simulink model "HvacFrntOutSurrndgsTOutl"  (R2022b)
settings: Solver=FixedStepDiscrete  StartTime=0.0  StopTime=10.0  FixedStep=0.2  RelTol=1e-3
settings Model: Creator=blue.ge  LastModifiedBy=blue.ge
settings DataIOCC: SaveFormat=StructureWithTime
settings OptimizationCC: DefaultParameterBehavior=Inlined  EnhancedBackFolding=on  PassReuseOutputArgsAs=Individual arguments  ZeroExternalMemoryAtStartup=off  ZeroInternalMemoryAtStartup=off  OptimizeBlockOrder=speed  DifferentSizesBufferReuse=on  OptimizationCustomize=off  EfficientTunableParamExpr=on
settings DebuggingCC: ParameterTunabilityLossMsg=error
settings ModelReferenceCC: ModelReferenceNumInstancesAllowed=Single
settings SFSimCC: MATLABDynamicMemAlloc=off
settings RTWCC: Description=AUTOSAR  SystemTargetFile=autosar.tlc  GenCodeOnly=on  TemplateMakefile=ert_default_tmf
settings CodeAppCC: ForceParamTrailComments=on  IgnoreCustomStorageClasses=off  OperatorAnnotations=on  SimulinkDataObjDesc=on  SFDataObjDesc=on  InsertBlockDesc=on
settings STFCustomTargetCC: TargetLangStandard=C99 (ISO)  MultiwordLength=256  ParMdlRefBuildCompliant=on  CompOptLevelCompliant=on  IncludeMdlTerminateFcn=off  CombineOutputUpdateFcns=on  SuppressErrorStatus=on  ERTCustomFileBanners=on  SupportNonFinite=off  SupportComplex=off  SupportContinuousTime=off  SupportNonInlinedSFcns=off  RemoveResetFunc=on  MATLABClassNameForMDSCustomization=Simulink.SoftwareTarget.ERTCustomization  AutosarCompliant=on  UseToolchainInfoCompliant=on  LookupTableObjectStructAxisOrder=2,1,3,4,...  LUTObjectStructOrderEvenSpacing=Size,Table,Breakpoints  SystemTargetFile=autosar.tlc
settings ERTTargetCC: TargetLangStandard=C99 (ISO)  ConcurrentExecutionCompliant=off  IncludeMdlTerminateFcn=off  SuppressErrorStatus=on  SupportNonFinite=off  SupportComplex=off  ModelStepFunctionPrototypeControlCompliant=off  CPPClassGenCompliant=off  AutosarCompliant=on  LookupTableObjectStructAxisOrder=2,1,3,4,...  LUTObjectStructOrderEvenSpacing=Size,Table,Breakpoints  InlinedParameterPlacement=NonHierarchical  ERTCustomFileTemplate=example_file_process.tlc

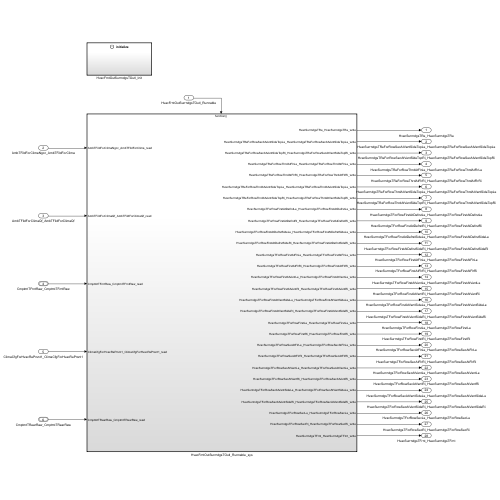
[thumbnail: Simulink's stored preview of the top-level diagram]
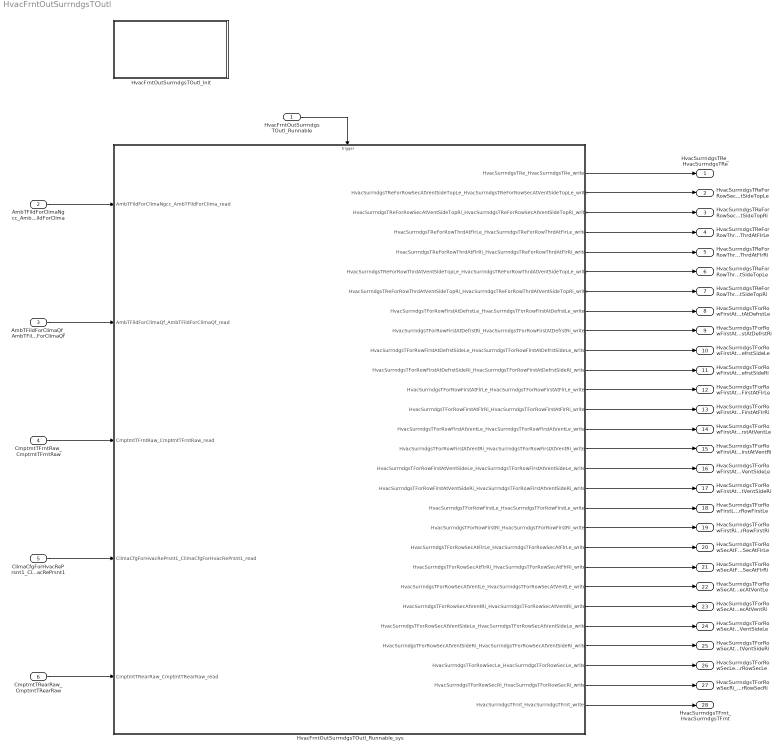
== system HvacFrntOutSurrndgsTOutl ==
  HvacFrntOutSurrndgsTOutl_Runnable : Inport  SampleTime=0.2  OutputFunctionCall=on
  AmbTFildForClimaNgcc_AmbTFildForClima : Inport  Port=2  OutDataTypeStr=T30  PortDimensions=1
  AmbTFildForClimaQf_AmbTFildForClimaQf : Inport  Port=3  OutDataTypeStr=Enum: GenQf1  PortDimensions=1
  CmptmtTFrntRaw_CmptmtTFrntRaw : Inport  Port=4  OutDataTypeStr=Bus: CmptmtTFrnt  PortDimensions=1  BusOutputAsStruct=on
  ClimaCfgForHvacRePrsnt1_ClimaCfgForHvacRePrsnt1 : Inport  Port=5  OutDataTypeStr=Enum: CarCfgForHvacRe  PortDimensions=1
  CmptmtTRearRaw_CmptmtTRearRaw : Inport  Port=6  OutDataTypeStr=Bus: CmptmtTFrnt  PortDimensions=1  BusOutputAsStruct=on
  HvacFrntOutSurrndgsTOutl_Init : Subsystem
  HvacFrntOutSurrndgsTOutl_Runnable_sys : Subsystem
  HvacSurrndgsTRe_HvacSurrndgsTRe : Outport  OutDataTypeStr=T30  PortDimensions=1
  HvacSurrndgsTReForRowSecAtVentSideTopLe_HvacSurrndgsTReForRowSecAtVentSideTopLe : Outport  Port=2  OutDataTypeStr=T30  PortDimensions=1
  HvacSurrndgsTReForRowSecAtVentSideTopRi_HvacSurrndgsTReForRowSecAtVentSideTopRi : Outport  Port=3  OutDataTypeStr=T30  PortDimensions=1
  HvacSurrndgsTReForRowThrdAtFlrLe_HvacSurrndgsTReForRowThrdAtFlrLe : Outport  Port=4  OutDataTypeStr=T30  PortDimensions=1
  HvacSurrndgsTReForRowThrdAtFlrRi_HvacSurrndgsTReForRowThrdAtFlrRi : Outport  Port=5  OutDataTypeStr=T30  PortDimensions=1
  HvacSurrndgsTReForRowThrdAtVentSideTopLe_HvacSurrndgsTReForRowThrdAtVentSideTopLe : Outport  Port=6  OutDataTypeStr=T30  PortDimensions=1
  HvacSurrndgsTReForRowThrdAtVentSideTopRi_HvacSurrndgsTReForRowThrdAtVentSideTopRi : Outport  Port=7  OutDataTypeStr=T30  PortDimensions=1
  HvacSurrndgsTForRowFirstAtDefrstLe_HvacSurrndgsTForRowFirstAtDefrstLe : Outport  Port=8  OutDataTypeStr=T30  PortDimensions=1
  HvacSurrndgsTForRowFirstAtDefrstRi_HvacSurrndgsTForRowFirstAtDefrstRi : Outport  Port=9  OutDataTypeStr=T30  PortDimensions=1
  HvacSurrndgsTForRowFirstAtDefrstSideLe_HvacSurrndgsTForRowFirstAtDefrstSideLe : Outport  Port=10  OutDataTypeStr=T30  PortDimensions=1
  HvacSurrndgsTForRowFirstAtDefrstSideRi_HvacSurrndgsTForRowFirstAtDefrstSideRi : Outport  Port=11  OutDataTypeStr=T30  PortDimensions=1
  HvacSurrndgsTForRowFirstAtFlrLe_HvacSurrndgsTForRowFirstAtFlrLe : Outport  Port=12  OutDataTypeStr=T30  PortDimensions=1
  HvacSurrndgsTForRowFirstAtFlrRi_HvacSurrndgsTForRowFirstAtFlrRi : Outport  Port=13  OutDataTypeStr=T30  PortDimensions=1
  HvacSurrndgsTForRowFirstAtVentLe_HvacSurrndgsTForRowFirstAtVentLe : Outport  Port=14  OutDataTypeStr=T30  PortDimensions=1
  HvacSurrndgsTForRowFirstAtVentRi_HvacSurrndgsTForRowFirstAtVentRi : Outport  Port=15  OutDataTypeStr=T30  PortDimensions=1
  HvacSurrndgsTForRowFirstAtVentSideLe_HvacSurrndgsTForRowFirstAtVentSideLe : Outport  Port=16  OutDataTypeStr=T30  PortDimensions=1
  HvacSurrndgsTForRowFirstAtVentSideRi_HvacSurrndgsTForRowFirstAtVentSideRi : Outport  Port=17  OutDataTypeStr=T30  PortDimensions=1
  HvacSurrndgsTForRowFirstLe_HvacSurrndgsTForRowFirstLe : Outport  Port=18  OutDataTypeStr=T30  PortDimensions=1
  HvacSurrndgsTForRowFirstRi_HvacSurrndgsTForRowFirstRi : Outport  Port=19  OutDataTypeStr=T30  PortDimensions=1
  HvacSurrndgsTForRowSecAtFlrLe_HvacSurrndgsTForRowSecAtFlrLe : Outport  Port=20  OutDataTypeStr=T30  PortDimensions=1
  HvacSurrndgsTForRowSecAtFlrRi_HvacSurrndgsTForRowSecAtFlrRi : Outport  Port=21  OutDataTypeStr=T30  PortDimensions=1
  HvacSurrndgsTForRowSecAtVentLe_HvacSurrndgsTForRowSecAtVentLe : Outport  Port=22  OutDataTypeStr=T30  PortDimensions=1
  HvacSurrndgsTForRowSecAtVentRi_HvacSurrndgsTForRowSecAtVentRi : Outport  Port=23  OutDataTypeStr=T30  PortDimensions=1
  HvacSurrndgsTForRowSecAtVentSideLe_HvacSurrndgsTForRowSecAtVentSideLe : Outport  Port=24  OutDataTypeStr=T30  PortDimensions=1
  HvacSurrndgsTForRowSecAtVentSideRi_HvacSurrndgsTForRowSecAtVentSideRi : Outport  Port=25  OutDataTypeStr=T30  PortDimensions=1
  HvacSurrndgsTForRowSecLe_HvacSurrndgsTForRowSecLe : Outport  Port=26  OutDataTypeStr=T30  PortDimensions=1
  HvacSurrndgsTForRowSecRi_HvacSurrndgsTForRowSecRi : Outport  Port=27  OutDataTypeStr=T30  PortDimensions=1
  HvacSurrndgsTFrnt_HvacSurrndgsTFrnt : Outport  Port=28  OutDataTypeStr=T30  PortDimensions=1
  wires:
  AmbTFildForClimaNgcc_AmbTFildForClima/1 -> HvacFrntOutSurrndgsTOutl_Runnable_sys/1(AmbTFildForClimaNgcc_AmbTFildForClima_read)
  AmbTFildForClimaQf_AmbTFildForClimaQf/1 -> HvacFrntOutSurrndgsTOutl_Runnable_sys/2(AmbTFildForClimaQf_AmbTFildForClimaQf_read)
  CmptmtTFrntRaw_CmptmtTFrntRaw/1 -> HvacFrntOutSurrndgsTOutl_Runnable_sys/3(CmptmtTFrntRaw_CmptmtTFrntRaw_read)
  ClimaCfgForHvacRePrsnt1_ClimaCfgForHvacRePrsnt1/1 -> HvacFrntOutSurrndgsTOutl_Runnable_sys/4(ClimaCfgForHvacRePrsnt1_ClimaCfgForHvacRePrsnt1_read)
  CmptmtTRearRaw_CmptmtTRearRaw/1 -> HvacFrntOutSurrndgsTOutl_Runnable_sys/5(CmptmtTRearRaw_CmptmtTRearRaw_read)
  HvacFrntOutSurrndgsTOutl_Runnable_sys/1(HvacSurrndgsTRe_HvacSurrndgsTRe_write) -> HvacSurrndgsTRe_HvacSurrndgsTRe/1
  HvacFrntOutSurrndgsTOutl_Runnable_sys/2(HvacSurrndgsTReForRowSecAtVentSideTopLe_HvacSurrndgsTReForRowSecAtVentSideTopLe_write) -> HvacSurrndgsTReForRowSecAtVentSideTopLe_HvacSurrndgsTReForRowSecAtVentSideTopLe/1
  HvacFrntOutSurrndgsTOutl_Runnable_sys/3(HvacSurrndgsTReForRowSecAtVentSideTopRi_HvacSurrndgsTReForRowSecAtVentSideTopRi_write) -> HvacSurrndgsTReForRowSecAtVentSideTopRi_HvacSurrndgsTReForRowSecAtVentSideTopRi/1
  HvacFrntOutSurrndgsTOutl_Runnable_sys/4(HvacSurrndgsTReForRowThrdAtFlrLe_HvacSurrndgsTReForRowThrdAtFlrLe_write) -> HvacSurrndgsTReForRowThrdAtFlrLe_HvacSurrndgsTReForRowThrdAtFlrLe/1
  HvacFrntOutSurrndgsTOutl_Runnable_sys/5(HvacSurrndgsTReForRowThrdAtFlrRi_HvacSurrndgsTReForRowThrdAtFlrRi_write) -> HvacSurrndgsTReForRowThrdAtFlrRi_HvacSurrndgsTReForRowThrdAtFlrRi/1
  HvacFrntOutSurrndgsTOutl_Runnable_sys/6(HvacSurrndgsTReForRowThrdAtVentSideTopLe_HvacSurrndgsTReForRowThrdAtVentSideTopLe_write) -> HvacSurrndgsTReForRowThrdAtVentSideTopLe_HvacSurrndgsTReForRowThrdAtVentSideTopLe/1
  HvacFrntOutSurrndgsTOutl_Runnable_sys/7(HvacSurrndgsTReForRowThrdAtVentSideTopRi_HvacSurrndgsTReForRowThrdAtVentSideTopRi_write) -> HvacSurrndgsTReForRowThrdAtVentSideTopRi_HvacSurrndgsTReForRowThrdAtVentSideTopRi/1
  HvacFrntOutSurrndgsTOutl_Runnable_sys/8(HvacSurrndgsTForRowFirstAtDefrstLe_HvacSurrndgsTForRowFirstAtDefrstLe_write) -> HvacSurrndgsTForRowFirstAtDefrstLe_HvacSurrndgsTForRowFirstAtDefrstLe/1
  HvacFrntOutSurrndgsTOutl_Runnable_sys/9(HvacSurrndgsTForRowFirstAtDefrstRi_HvacSurrndgsTForRowFirstAtDefrstRi_write) -> HvacSurrndgsTForRowFirstAtDefrstRi_HvacSurrndgsTForRowFirstAtDefrstRi/1
  HvacFrntOutSurrndgsTOutl_Runnable_sys/10(HvacSurrndgsTForRowFirstAtDefrstSideLe_HvacSurrndgsTForRowFirstAtDefrstSideLe_write) -> HvacSurrndgsTForRowFirstAtDefrstSideLe_HvacSurrndgsTForRowFirstAtDefrstSideLe/1
  HvacFrntOutSurrndgsTOutl_Runnable_sys/11(HvacSurrndgsTForRowFirstAtDefrstSideRi_HvacSurrndgsTForRowFirstAtDefrstSideRi_write) -> HvacSurrndgsTForRowFirstAtDefrstSideRi_HvacSurrndgsTForRowFirstAtDefrstSideRi/1
  HvacFrntOutSurrndgsTOutl_Runnable_sys/12(HvacSurrndgsTForRowFirstAtFlrLe_HvacSurrndgsTForRowFirstAtFlrLe_write) -> HvacSurrndgsTForRowFirstAtFlrLe_HvacSurrndgsTForRowFirstAtFlrLe/1
  HvacFrntOutSurrndgsTOutl_Runnable_sys/13(HvacSurrndgsTForRowFirstAtFlrRi_HvacSurrndgsTForRowFirstAtFlrRi_write) -> HvacSurrndgsTForRowFirstAtFlrRi_HvacSurrndgsTForRowFirstAtFlrRi/1
  HvacFrntOutSurrndgsTOutl_Runnable_sys/14(HvacSurrndgsTForRowFirstAtVentLe_HvacSurrndgsTForRowFirstAtVentLe_write) -> HvacSurrndgsTForRowFirstAtVentLe_HvacSurrndgsTForRowFirstAtVentLe/1
  HvacFrntOutSurrndgsTOutl_Runnable_sys/15(HvacSurrndgsTForRowFirstAtVentRi_HvacSurrndgsTForRowFirstAtVentRi_write) -> HvacSurrndgsTForRowFirstAtVentRi_HvacSurrndgsTForRowFirstAtVentRi/1
  HvacFrntOutSurrndgsTOutl_Runnable_sys/16(HvacSurrndgsTForRowFirstAtVentSideLe_HvacSurrndgsTForRowFirstAtVentSideLe_write) -> HvacSurrndgsTForRowFirstAtVentSideLe_HvacSurrndgsTForRowFirstAtVentSideLe/1
  HvacFrntOutSurrndgsTOutl_Runnable_sys/17(HvacSurrndgsTForRowFirstAtVentSideRi_HvacSurrndgsTForRowFirstAtVentSideRi_write) -> HvacSurrndgsTForRowFirstAtVentSideRi_HvacSurrndgsTForRowFirstAtVentSideRi/1
  HvacFrntOutSurrndgsTOutl_Runnable_sys/18(HvacSurrndgsTForRowFirstLe_HvacSurrndgsTForRowFirstLe_write) -> HvacSurrndgsTForRowFirstLe_HvacSurrndgsTForRowFirstLe/1
  HvacFrntOutSurrndgsTOutl_Runnable_sys/19(HvacSurrndgsTForRowFirstRi_HvacSurrndgsTForRowFirstRi_write) -> HvacSurrndgsTForRowFirstRi_HvacSurrndgsTForRowFirstRi/1
  HvacFrntOutSurrndgsTOutl_Runnable_sys/20(HvacSurrndgsTForRowSecAtFlrLe_HvacSurrndgsTForRowSecAtFlrLe_write) -> HvacSurrndgsTForRowSecAtFlrLe_HvacSurrndgsTForRowSecAtFlrLe/1
  HvacFrntOutSurrndgsTOutl_Runnable_sys/21(HvacSurrndgsTForRowSecAtFlrRi_HvacSurrndgsTForRowSecAtFlrRi_write) -> HvacSurrndgsTForRowSecAtFlrRi_HvacSurrndgsTForRowSecAtFlrRi/1
  HvacFrntOutSurrndgsTOutl_Runnable_sys/22(HvacSurrndgsTForRowSecAtVentLe_HvacSurrndgsTForRowSecAtVentLe_write) -> HvacSurrndgsTForRowSecAtVentLe_HvacSurrndgsTForRowSecAtVentLe/1
  HvacFrntOutSurrndgsTOutl_Runnable_sys/23(HvacSurrndgsTForRowSecAtVentRi_HvacSurrndgsTForRowSecAtVentRi_write) -> HvacSurrndgsTForRowSecAtVentRi_HvacSurrndgsTForRowSecAtVentRi/1
  HvacFrntOutSurrndgsTOutl_Runnable_sys/24(HvacSurrndgsTForRowSecAtVentSideLe_HvacSurrndgsTForRowSecAtVentSideLe_write) -> HvacSurrndgsTForRowSecAtVentSideLe_HvacSurrndgsTForRowSecAtVentSideLe/1
  HvacFrntOutSurrndgsTOutl_Runnable_sys/25(HvacSurrndgsTForRowSecAtVentSideRi_HvacSurrndgsTForRowSecAtVentSideRi_write) -> HvacSurrndgsTForRowSecAtVentSideRi_HvacSurrndgsTForRowSecAtVentSideRi/1
  HvacFrntOutSurrndgsTOutl_Runnable_sys/26(HvacSurrndgsTForRowSecLe_HvacSurrndgsTForRowSecLe_write) -> HvacSurrndgsTForRowSecLe_HvacSurrndgsTForRowSecLe/1
  HvacFrntOutSurrndgsTOutl_Runnable_sys/27(HvacSurrndgsTForRowSecRi_HvacSurrndgsTForRowSecRi_write) -> HvacSurrndgsTForRowSecRi_HvacSurrndgsTForRowSecRi/1
  HvacFrntOutSurrndgsTOutl_Runnable_sys/28(HvacSurrndgsTFrnt_HvacSurrndgsTFrnt_write) -> HvacSurrndgsTFrnt_HvacSurrndgsTFrnt/1
  HvacFrntOutSurrndgsTOutl_Runnable/1 -> HvacFrntOutSurrndgsTOutl_Runnable_sys/Trigger

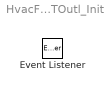
== system HvacFrntOutSurrndgsTOutl/HvacFrntOutSurrndgsTOutl_Init ==
  Event Listener : EventListener  EventName=reset

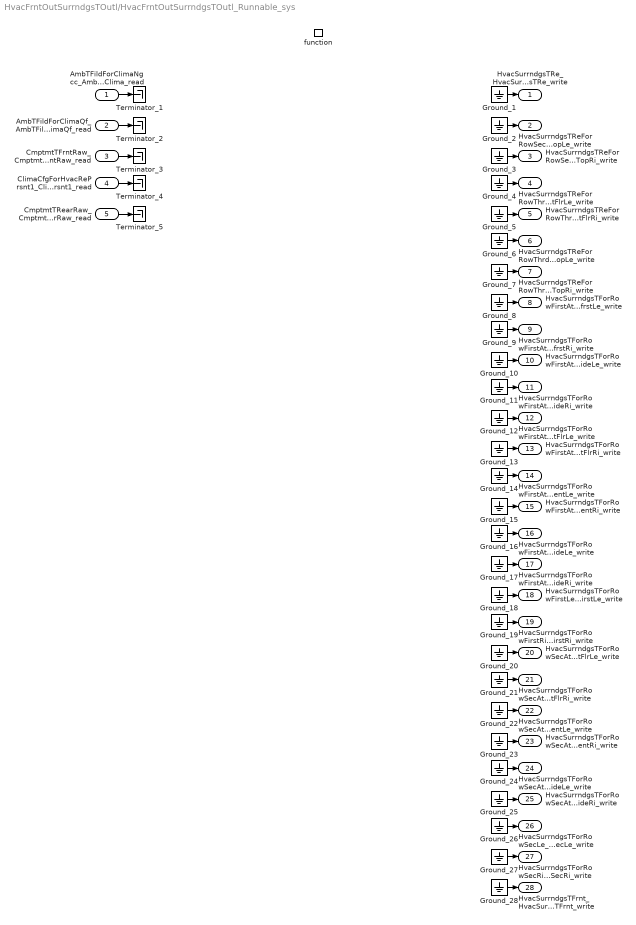
== system HvacFrntOutSurrndgsTOutl/HvacFrntOutSurrndgsTOutl_Runnable_sys ==
  AmbTFildForClimaNgcc_AmbTFildForClima_read : Inport
  AmbTFildForClimaQf_AmbTFildForClimaQf_read : Inport  Port=2
  CmptmtTFrntRaw_CmptmtTFrntRaw_read : Inport  Port=3
  ClimaCfgForHvacRePrsnt1_ClimaCfgForHvacRePrsnt1_read : Inport  Port=4
  CmptmtTRearRaw_CmptmtTRearRaw_read : Inport  Port=5
  function : TriggerPort  TriggerType=function-call  VariantControl=(inherit)  SampleTimeType=periodic  SampleTime=-1
  Ground_{1..28} (28 alike) : Ground
  Terminator_{1..5} (5 alike) : Terminator
  HvacSurrndgsTRe_HvacSurrndgsTRe_write : Outport
      settings: EnsureOutportIsVirtual=on
  HvacSurrndgsTReForRowSecAtVentSideTopLe_HvacSurrndgsTReForRowSecAtVentSideTopLe_write : Outport  Port=2
      settings: EnsureOutportIsVirtual=on
  HvacSurrndgsTReForRowSecAtVentSideTopRi_HvacSurrndgsTReForRowSecAtVentSideTopRi_write : Outport  Port=3
      settings: EnsureOutportIsVirtual=on
  HvacSurrndgsTReForRowThrdAtFlrLe_HvacSurrndgsTReForRowThrdAtFlrLe_write : Outport  Port=4
      settings: EnsureOutportIsVirtual=on
  HvacSurrndgsTReForRowThrdAtFlrRi_HvacSurrndgsTReForRowThrdAtFlrRi_write : Outport  Port=5
      settings: EnsureOutportIsVirtual=on
  HvacSurrndgsTReForRowThrdAtVentSideTopLe_HvacSurrndgsTReForRowThrdAtVentSideTopLe_write : Outport  Port=6
      settings: EnsureOutportIsVirtual=on
  HvacSurrndgsTReForRowThrdAtVentSideTopRi_HvacSurrndgsTReForRowThrdAtVentSideTopRi_write : Outport  Port=7
      settings: EnsureOutportIsVirtual=on
  HvacSurrndgsTForRowFirstAtDefrstLe_HvacSurrndgsTForRowFirstAtDefrstLe_write : Outport  Port=8
      settings: EnsureOutportIsVirtual=on
  HvacSurrndgsTForRowFirstAtDefrstRi_HvacSurrndgsTForRowFirstAtDefrstRi_write : Outport  Port=9
      settings: EnsureOutportIsVirtual=on
  HvacSurrndgsTForRowFirstAtDefrstSideLe_HvacSurrndgsTForRowFirstAtDefrstSideLe_write : Outport  Port=10
      settings: EnsureOutportIsVirtual=on
  HvacSurrndgsTForRowFirstAtDefrstSideRi_HvacSurrndgsTForRowFirstAtDefrstSideRi_write : Outport  Port=11
      settings: EnsureOutportIsVirtual=on
  HvacSurrndgsTForRowFirstAtFlrLe_HvacSurrndgsTForRowFirstAtFlrLe_write : Outport  Port=12
      settings: EnsureOutportIsVirtual=on
  HvacSurrndgsTForRowFirstAtFlrRi_HvacSurrndgsTForRowFirstAtFlrRi_write : Outport  Port=13
      settings: EnsureOutportIsVirtual=on
  HvacSurrndgsTForRowFirstAtVentLe_HvacSurrndgsTForRowFirstAtVentLe_write : Outport  Port=14
      settings: EnsureOutportIsVirtual=on
  HvacSurrndgsTForRowFirstAtVentRi_HvacSurrndgsTForRowFirstAtVentRi_write : Outport  Port=15
      settings: EnsureOutportIsVirtual=on
  HvacSurrndgsTForRowFirstAtVentSideLe_HvacSurrndgsTForRowFirstAtVentSideLe_write : Outport  Port=16
      settings: EnsureOutportIsVirtual=on
  HvacSurrndgsTForRowFirstAtVentSideRi_HvacSurrndgsTForRowFirstAtVentSideRi_write : Outport  Port=17
      settings: EnsureOutportIsVirtual=on
  HvacSurrndgsTForRowFirstLe_HvacSurrndgsTForRowFirstLe_write : Outport  Port=18
      settings: EnsureOutportIsVirtual=on
  HvacSurrndgsTForRowFirstRi_HvacSurrndgsTForRowFirstRi_write : Outport  Port=19
      settings: EnsureOutportIsVirtual=on
  HvacSurrndgsTForRowSecAtFlrLe_HvacSurrndgsTForRowSecAtFlrLe_write : Outport  Port=20
      settings: EnsureOutportIsVirtual=on
  HvacSurrndgsTForRowSecAtFlrRi_HvacSurrndgsTForRowSecAtFlrRi_write : Outport  Port=21
      settings: EnsureOutportIsVirtual=on
  HvacSurrndgsTForRowSecAtVentLe_HvacSurrndgsTForRowSecAtVentLe_write : Outport  Port=22
      settings: EnsureOutportIsVirtual=on
  HvacSurrndgsTForRowSecAtVentRi_HvacSurrndgsTForRowSecAtVentRi_write : Outport  Port=23
      settings: EnsureOutportIsVirtual=on
  HvacSurrndgsTForRowSecAtVentSideLe_HvacSurrndgsTForRowSecAtVentSideLe_write : Outport  Port=24
      settings: EnsureOutportIsVirtual=on
  HvacSurrndgsTForRowSecAtVentSideRi_HvacSurrndgsTForRowSecAtVentSideRi_write : Outport  Port=25
      settings: EnsureOutportIsVirtual=on
  HvacSurrndgsTForRowSecLe_HvacSurrndgsTForRowSecLe_write : Outport  Port=26
      settings: EnsureOutportIsVirtual=on
  HvacSurrndgsTForRowSecRi_HvacSurrndgsTForRowSecRi_write : Outport  Port=27
      settings: EnsureOutportIsVirtual=on
  HvacSurrndgsTFrnt_HvacSurrndgsTFrnt_write : Outport  Port=28
      settings: EnsureOutportIsVirtual=on
  wires:
  AmbTFildForClimaNgcc_AmbTFildForClima_read/1 -> Terminator_1/1
  AmbTFildForClimaQf_AmbTFildForClimaQf_read/1 -> Terminator_2/1
  CmptmtTFrntRaw_CmptmtTFrntRaw_read/1 -> Terminator_3/1
  ClimaCfgForHvacRePrsnt1_ClimaCfgForHvacRePrsnt1_read/1 -> Terminator_4/1
  CmptmtTRearRaw_CmptmtTRearRaw_read/1 -> Terminator_5/1
  Ground_1/1 -> HvacSurrndgsTRe_HvacSurrndgsTRe_write/1
  Ground_2/1 -> HvacSurrndgsTReForRowSecAtVentSideTopLe_HvacSurrndgsTReForRowSecAtVentSideTopLe_write/1
  Ground_3/1 -> HvacSurrndgsTReForRowSecAtVentSideTopRi_HvacSurrndgsTReForRowSecAtVentSideTopRi_write/1
  Ground_4/1 -> HvacSurrndgsTReForRowThrdAtFlrLe_HvacSurrndgsTReForRowThrdAtFlrLe_write/1
  Ground_5/1 -> HvacSurrndgsTReForRowThrdAtFlrRi_HvacSurrndgsTReForRowThrdAtFlrRi_write/1
  Ground_6/1 -> HvacSurrndgsTReForRowThrdAtVentSideTopLe_HvacSurrndgsTReForRowThrdAtVentSideTopLe_write/1
  Ground_7/1 -> HvacSurrndgsTReForRowThrdAtVentSideTopRi_HvacSurrndgsTReForRowThrdAtVentSideTopRi_write/1
  Ground_8/1 -> HvacSurrndgsTForRowFirstAtDefrstLe_HvacSurrndgsTForRowFirstAtDefrstLe_write/1
  Ground_9/1 -> HvacSurrndgsTForRowFirstAtDefrstRi_HvacSurrndgsTForRowFirstAtDefrstRi_write/1
  Ground_10/1 -> HvacSurrndgsTForRowFirstAtDefrstSideLe_HvacSurrndgsTForRowFirstAtDefrstSideLe_write/1
  Ground_11/1 -> HvacSurrndgsTForRowFirstAtDefrstSideRi_HvacSurrndgsTForRowFirstAtDefrstSideRi_write/1
  Ground_12/1 -> HvacSurrndgsTForRowFirstAtFlrLe_HvacSurrndgsTForRowFirstAtFlrLe_write/1
  Ground_13/1 -> HvacSurrndgsTForRowFirstAtFlrRi_HvacSurrndgsTForRowFirstAtFlrRi_write/1
  Ground_14/1 -> HvacSurrndgsTForRowFirstAtVentLe_HvacSurrndgsTForRowFirstAtVentLe_write/1
  Ground_15/1 -> HvacSurrndgsTForRowFirstAtVentRi_HvacSurrndgsTForRowFirstAtVentRi_write/1
  Ground_16/1 -> HvacSurrndgsTForRowFirstAtVentSideLe_HvacSurrndgsTForRowFirstAtVentSideLe_write/1
  Ground_17/1 -> HvacSurrndgsTForRowFirstAtVentSideRi_HvacSurrndgsTForRowFirstAtVentSideRi_write/1
  Ground_18/1 -> HvacSurrndgsTForRowFirstLe_HvacSurrndgsTForRowFirstLe_write/1
  Ground_19/1 -> HvacSurrndgsTForRowFirstRi_HvacSurrndgsTForRowFirstRi_write/1
  Ground_20/1 -> HvacSurrndgsTForRowSecAtFlrLe_HvacSurrndgsTForRowSecAtFlrLe_write/1
  Ground_21/1 -> HvacSurrndgsTForRowSecAtFlrRi_HvacSurrndgsTForRowSecAtFlrRi_write/1
  Ground_22/1 -> HvacSurrndgsTForRowSecAtVentLe_HvacSurrndgsTForRowSecAtVentLe_write/1
  Ground_23/1 -> HvacSurrndgsTForRowSecAtVentRi_HvacSurrndgsTForRowSecAtVentRi_write/1
  Ground_24/1 -> HvacSurrndgsTForRowSecAtVentSideLe_HvacSurrndgsTForRowSecAtVentSideLe_write/1
  Ground_25/1 -> HvacSurrndgsTForRowSecAtVentSideRi_HvacSurrndgsTForRowSecAtVentSideRi_write/1
  Ground_26/1 -> HvacSurrndgsTForRowSecLe_HvacSurrndgsTForRowSecLe_write/1
  Ground_27/1 -> HvacSurrndgsTForRowSecRi_HvacSurrndgsTForRowSecRi_write/1
  Ground_28/1 -> HvacSurrndgsTFrnt_HvacSurrndgsTFrnt_write/1
